simulink model "FlightSim"  (R2023a)
settings: Solver=VariableStepAuto  StartTime=0.0  StopTime=20  RelTol=1e-3
model workspace (MAT data in the model file):
  polyCoefficient = [1 1 1]
values from neighbouring files (symbols this model uses):
  airDensity = 1.2250    (= 1.225)    [FlightSimSetup.m]
  airKinematicViscosity = 1.48*10^-5    (= 1.48e-05)    [FlightSimSetup.m]
  AOA = deg2rad(0)    [FlightSimSetup.m]
  axialDistanceMACCGx = abs(finMACLeadingEdgeLocation) + finLeadingEdgeCGxlength    [finParameters.m]
  bodyDiameter = 2/100    (= 0.02)    [FlightSimSetup.m]
  bodyLength = 20/100    (= 0.2)    [FlightSimSetup.m]
  bodyLongitudinalInertia = bodyMass*( ((bodyDiameter/2).^2)/4 + ((bodyLength).^2)/12)    [InertiaParameters.m]
  bodyMass = 12/1000    (= 0.012)    [FlightSimSetup.m]
  bodyPlanformArea = bodyDiameter*bodyLength    (= 0.004)    [FlightSimSetup.m]
  bodyRoughness = 60*10^-6    (= 6e-05)    [FlightSimSetup.m]
  casingLongitudinalInertia = motorCasingMass/12*(3*(motorOuterRadius.^2+motorInnerRadius.^2)+motorLength.^2)    [InertiaParameters.m]
  cYaw = 0    [FlightSimSetup.m]
  dIdt = zeros(3)    [InertiaParameters.m]
  finArea = (finRootChord+finTailChord)*finSpan/2    [finParameters.m]
  finCantAngle = deg2rad(0)    [FlightSimSetup.m]
  finCentroidAxial = finSpan/3 * ( (finRootChord + 2*finTailChord)/(finRootChord + finTailChord) ) - backOffset    [finParameters.m]
  finCount = 4    [FlightSimSetup.m]
  finFrontSweepAngle = acos(dot(frontSweepAngleRefVector1, frontSweepAngleRefVector2) / (norm(frontSweepAngleRefVector1) * norm(frontSweepAngleRefVector2)))    [finParameters.m]
  finLeadingEdgeNoseConeDistance = bodyLength + noseConeHeight - finRootChord + backOffset    [finParameters.m]
  finLongitudinalInertia = finLongitudinalInertia * singleFinMass * finCount    [InertiaParameters.m]
  finMACLeadingEdgeLocation = abs( integral(finMACLeadingEdgeLocationFunction, 0, finSpan)/finArea )    [finParameters.m]
  finMACLength = integral(chordLengthFunction, 0, finSpan)/finArea    [finParameters.m]
  finMACNoseconeAxialDistance = finLeadingEdgeNoseConeDistance + finMACLeadingEdgeLocation+ 1/2*finMACLength    [finParameters.m]
  finMidChordSweepAngle = acos(dot(midChordAngleRefVector1, midChordAngleRefVector2) / (norm(midChordAngleRefVector1) * norm(midChordAngleRefVector2)))    [finParameters.m]
  finProfileType = 3    [FlightSimSetup.m]
  finRootChord = abs(P_frontRoot(2)-P_backRoot(2))    [finParameters.m]
  finSpan = abs(P_frontTrail(1)- P_frontRoot(1))    [finParameters.m]
  finTailChord = abs(P_frontTrail(2)-P_backTrail(2))    [finParameters.m]
  finThickness = 0.15/100    (= 0.0015)    [FlightSimSetup.m]
  finX_t = P_frontRoot(2) - P_frontTrail(2)    [finParameters.m]
  launchLugInnerDiameter = 0    [FlightSimSetup.m]
  launchLugLength = 0    [FlightSimSetup.m]
  launchLugOuterDiameter = 0    [FlightSimSetup.m]
  machNum = 0    [FlightSimSetup.m]
  motorCasingMass = motorInitialTotalMass - motorInitialPropMass    (= 0.071)    [FlightSimSetup.m]
  motorInitialPropMass = 83/1000    (= 0.083)    [FlightSimSetup.m]
  motorInnerRadius = motorOuterRadius - casingThickness    [InertiaParameters.m]
  motorLength = 156/1000    (= 0.156)    [FlightSimSetup.m]
  motorTotalImpulse = 129.9    [FlightSimSetup.m]
  noseConeHeight = 6/100    (= 0.06)    [FlightSimSetup.m]
  noseConeJoinAngle = pi/2 - atan(noseConeHeight/(bodyDiameter/2))    [FlightSimSetup.m]
  noseConeLongitudinalInertia = noseConeLongitudinalInertia*noseConeMass    [noseConeParameters.m]
  noseConeMass = 5/1000    (= 0.005)    [FlightSimSetup.m]
  noseConePlanformArea = (1/2)*bodyDiameter* noseConeHeight    [noseConeParameters.m]
  noseConeType = 8    [FlightSimSetup.m]
  noseConeVolume = (1/3)*noseConeHeight*(bodyDiameter/2)^2*pi    [noseConeParameters.m]
  noseConeWettedArea = pi*sqrt( (bodyDiameter/2)^2 + noseConeHeight^2)*bodyDiameter/2    [noseConeParameters.m]
  pitchCenterX = 0    [FlightSimSetup.m]
  pitchRate = 0    [FlightSimSetup.m]
  radialMACPosition = finMACSpanwisePosition + bodyDiameter/2    [finParameters.m]
  refArea = bodyDiameter^2*pi/4    (= 0.000314159265359)    [FlightSimSetup.m]
  rocketLength = (20+6)/100    (= 0.26)    [FlightSimSetup.m]
  rocketNonMotorMass = noseConeMass + bodyMass + totalFinMass    (= 0.021)    [FlightSimSetup.m]
  rocketRotationalInertia = bodyRotationalInertia + casingRotationalInertia + finRotationalInertia + noseConeLongitudinalInertia    [InertiaParameters.m]
  rocketVelocityMag = machNum*340.17    (= 0)    [FlightSimSetup.m]
  rollRate = 0    [FlightSimSetup.m]
  totalFinMass = singleFinMass * finCount    (= 0.004)    [FlightSimSetup.m]
  windAngle = 0    [FlightSimSetup.m]
  yawRate = 0    [FlightSimSetup.m]
libraries: shared6dof x1, aerolibanim x1, aerolibadyn x1, aerolibbdyn x1, sharedutil x1
(part 2 of 3)

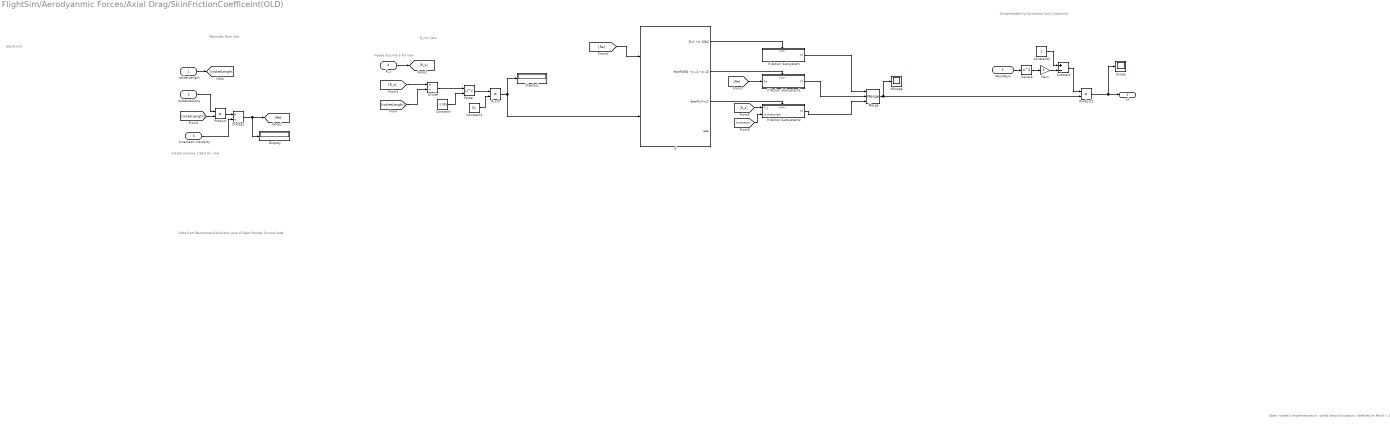
== system FlightSim/Aerodyanmic Forces/Axial Drag/SkinFrictionCoefficeint(OLD) ==
  note: Open rocket's implementation, works beyond subsonic (defined at Mach <.9)
  note: Code from BarrowmanCalculator.java of Open Rocket Source Code
  note: maybe assume 5 for now
  note: equations
  note: R_crit Calc
  note: Reynolds Num Calc
  note: maybe assume 1.5E-5 for now
  note: Compressability Correction (only Subsonic)
  rocketLength : Inport
  rocketVelocity : Inport  Port=2
  kinematic Viscosity : Inport  Port=3
  R_s : Inport  Port=4
  MachNum : Inport  Port=5
  Constant : Constant  Value=-1.039
  Constant1 : Constant  Value=51
  Constant2 : Constant
  Display : Display
  Display1 : Display
  Divide : Product  Inputs=*/
  Divide1 : Product  Inputs=*/
  Gain : Gain  Gain=.1
  If : If  NumInputs=2  IfExpression=u1 <= 10E4  ElseIfExpressions="10E4 <= u1 <= u2,  u1>u2"
  If Action Subsystem : Subsystem  TreatAsAtomicUnit=on
  If Action Subsystem1 : Subsystem  TreatAsAtomicUnit=on
  If Action Subsystem2 : Subsystem  TreatAsAtomicUnit=on
  Merge : Merge  Inputs=3
  Power : Math  Operator=pow
  Product : Product
  Product1 : Product
  R_crit : Product
  Scope : Scope
  Square : Math  Operator=square
  Subtract : Sum  Inputs=+-
  cfScope : Scope
  Cf : Outport
  wires:
  [R_s] -> Divide/1
  Divide/1 -> Power/1
  Constant/1 -> Power/2
  Power/1 -> R_crit/1
  Constant1/1 -> R_crit/2
  rocketLength/1 -> [rocketLength]
  R_crit/1 -> Display1/1, If/2
  [rocketLength] -> Divide/2
  [rocketLength] -> Product/2
  rocketVelocity/1 -> Product/1
  Product/1 -> Divide1/1
  kinematic Viscosity/1 -> Divide1/2
  Divide1/1 -> Display/1, [Re]
  R_s/1 -> [R_s]
  [Re] -> If/1
  If/1 -> If Action Subsystem/Action
  If/2 -> If Action Subsystem1/Action
  If Action Subsystem1/1(Cf) -> Merge/2
  If/3 -> If Action Subsystem2/Action
  If Action Subsystem2/1(Cf) -> Merge/3
  If Action Subsystem/1(Cf) -> Merge/1
  Merge/1 -> cfScope/1, Product1/2
  [Re] -> If Action Subsystem1/1(Re)
  [R_s] -> If Action Subsystem2/1(R_s)
  [rocketLength] -> If Action Subsystem2/2(rocketLength)
  MachNum/1 -> Square/1
  Square/1 -> Gain/1
  Gain/1 -> Subtract/2
  Constant2/1 -> Subtract/1
  Subtract/1 -> Product1/1
  Product1/1 -> Scope/1, Cf/1

== system FlightSim/Aerodyanmic Forces/Axial Drag/SkinFrictionCoefficient ==
  note: Open rocket's implementation, works beyond subsonic (defined at Mach <.9)
  note: Code from BarrowmanCalculator.java of Open Rocket Source Code
  note: equations
  note: Reynolds Num Calc
  note: maybe assume 1.5E-5 for now
  note: Compressability Correction (only Subsonic)
  note: Code from BarrowmanCalculator.java of Open Rocket Source Code, lines 601 to 632
  note: Roughness Comparison Body (Cf the same for Body, Fin, and nosecone)
  note: Code from BarrowmanCalculator.java of Open Rocket Source Code, lines 462-466
  rocketLength : Inport
  rocketVelocity : Inport  Port=2
  kinematic Viscosity : Inport  Port=3
  R_s : Inport  Port=4
  MachNum : Inport  Port=5
  Cf Value : Display
  Cf(R>R_Crit) : Product
  Constant : Constant  Value=1.48e-2
  Constant1 : Constant  Value=5.6
  Constant2 : Constant
  Constant3 : Constant
  Constant4 : Constant  Value=.2
  Constant5 : Constant  Value=0.032
  Display1 : Display
  Divide1 : Product  Inputs=*/
  Divide2 : Product  Inputs=*/
  Divide3 : Product  Inputs=*/
  Gain : Gain  Gain=.1
  Gain1 : Gain  Gain=1.5
  Log : Math  Operator=log
  Max : MinMax  Function=max  Inputs=2
  Power1 : Math  Operator=pow
  Product : Product
  Product1 : Product
  Product2 : Product
  Square : Math  Operator=square
  Square1 : Math  Operator=square
  Subtract : Sum  Inputs=+-
  Subtract1 : Sum  Inputs=+-
  Switch : Switch  Criteria=u2 > Threshold  Threshold=1e4  SaturateOnIntegerOverflow=off  InputSameDT=off
  reynolds Number : Display
  roughnessCorrection : Scope
  roughnessLimitedBody : Display
  Cf : Outport
  wires:
  rocketLength/1 -> [rocketLength]
  [rocketLength] -> Product/2
  rocketVelocity/1 -> Product/1
  Product/1 -> Divide1/1
  kinematic Viscosity/1 -> Divide1/2
  Divide1/1 -> Log/1, Switch/2, reynolds Number/1
  Switch/1 -> Product1/2
  MachNum/1 -> Square/1
  Square/1 -> Gain/1
  Gain/1 -> Subtract/2
  Constant2/1 -> Subtract/1
  Product1/1 -> Max/2, Cf Value/1
  Subtract1/1 -> Square1/1
  Constant1/1 -> Subtract1/2
  Square1/1 -> Divide2/2
  Log/1 -> Gain1/1
  Gain1/1 -> Subtract1/1
  Constant3/1 -> Divide2/1
  Constant/1 -> Switch/3
  Divide3/1 -> Power1/1
  Power1/1 -> Cf(R>R_Crit)/1
  Constant4/1 -> Power1/2
  Constant5/1 -> Cf(R>R_Crit)/2
  R_s/1 -> Divide3/1
  Cf(R>R_Crit)/1 -> Product2/1
  Subtract/1 -> Product1/1, roughnessCorrection/1, Product2/2
  Product2/1 -> Max/1, roughnessLimitedBody/1
  Max/1 -> Display1/1, Cf/1
  Divide2/1 -> Switch/1
  [rocketLength] -> Divide3/2

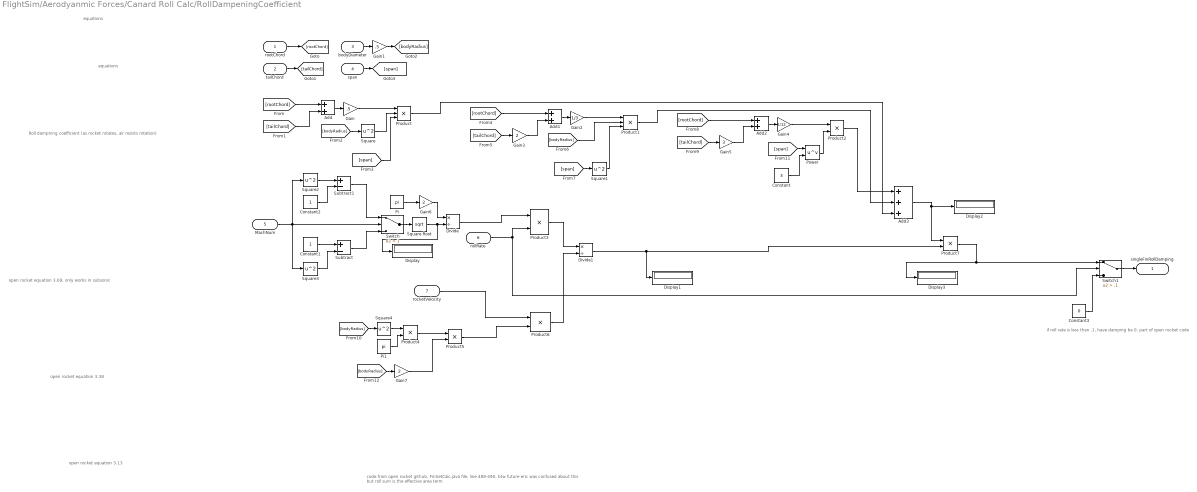
== system FlightSim/Aerodyanmic Forces/Canard Roll Calc/RollDampeningCoefficient ==
  note: if roll rate is less than .1, have damping be 0, part of open rocket code
  note: code from open rocket github, FinSetCalc.java file, line 488-490, btw future eric was confused about this but roll sum is the effective area term
  note: open rocket equation 3.38
  note: Roll dampning coefficient (as rocket rotates, air resists rotation)
  note: equations
  note: equations
  note: open rocket equation 3.13
  note: open rocket equation 3.69, only works in subsonic
  rootChord : Inport
  tailChord : Inport  Port=2
  bodyDiameter : Inport  Port=3
  span : Inport  Port=4
  MachNum : Inport  Port=5
  rollRate : Inport  Port=6
  rocketVelocity : Inport  Port=7
  Add : Sum
  Add1 : Sum
  Add2 : Sum
  Add3 : Sum  Inputs=+++
  Constant : Constant  Value=3
  Constant1 : Constant
  Constant2 : Constant
  Constant3 : Constant  Value=0
  Display{,1..3} (4 alike) : Display
  Divide : Product  Inputs=*/
  Divide1 : Product  Inputs=*/
  Gain : Gain  Gain=.5
  Gain1 : Gain  Gain=.5
  Gain2 : Gain  Gain=1/3
  Gain3 : Gain  Gain=2
  Gain4 : Gain  Gain=1/12
  Gain5 : Gain  Gain=3
  Gain6 : Gain  Gain=2
  Gain7 : Gain  Gain=2
  Pi : Constant  Value=pi  OutDataTypeStr=Inherit: Inherit via back propagation
  Pi1 : Constant  Value=pi  OutDataTypeStr=Inherit: Inherit via back propagation
  Power : Math  Operator=pow
  Product : Product  Inputs=3
  Product1 : Product  Inputs=3
  Product{2..7} (6 alike) : Product
  Square{,1..4} (5 alike) : Math  Operator=square
  Square Root : Sqrt
  Subtract : Sum  Inputs=+-
  Subtract1 : Sum  Inputs=+-
  Switch : Switch  Criteria=u2 > Threshold  Threshold=1  SaturateOnIntegerOverflow=off  InputSameDT=off
  Switch1 : Switch  Criteria=u2 > Threshold  Threshold=.1  SaturateOnIntegerOverflow=off  InputSameDT=off
  singleFinRollDamping : Outport
  wires:
  Add/1 -> Gain/1
  rootChord/1 -> [rootChord]
  tailChord/1 -> [tailChord]
  bodyDiameter/1 -> Gain1/1
  span/1 -> [span]
  [rootChord] -> Add/1
  [tailChord] -> Add/2
  Gain/1 -> Product/1
  Gain1/1 -> [bodyRadius]
  [bodyRadius] -> Square/1
  Square/1 -> Product/2
  [span] -> Product/3
  [tailChord] -> Gain3/1
  [rootChord] -> Add1/1
  Add1/1 -> Gain2/1
  Gain2/1 -> Product1/1
  Gain3/1 -> Add1/2
  [span] -> Square1/1
  Square1/1 -> Product1/3
  [bodyRadius] -> Product1/2
  [span] -> Power/1
  [tailChord] -> Gain5/1
  Gain5/1 -> Add2/2
  [rootChord] -> Add2/1
  Add2/1 -> Gain4/1
  Gain4/1 -> Product2/1
  Constant/1 -> Power/2
  Power/1 -> Product2/2
  Product2/1 -> Add3/1
  Product1/1 -> Add3/2
  Product/1 -> Add3/3
  Add3/1 -> Display2/1, Product7/1
  MachNum/1 -> Switch/2, Square3/1, Square2/1
  Constant1/1 -> Subtract/1
  Square3/1 -> Subtract/2
  Subtract/1 -> Switch/3
  Constant2/1 -> Subtract1/2
  Square2/1 -> Subtract1/1
  Subtract1/1 -> Switch/1
  rollRate/1 -> Product3/2, Switch1/2
  Pi/1 -> Gain6/1
  Gain6/1 -> Divide/1
  Divide/1 -> Product3/1
  [bodyRadius] -> Square4/1
  Square4/1 -> Product4/1
  Pi1/1 -> Product4/2
  Product4/1 -> Product5/1
  [bodyRadius] -> Gain7/1
  Gain7/1 -> Product5/2
  Product5/1 -> Product6/2
  rocketVelocity/1 -> Product6/1
  Product3/1 -> Divide1/1
  Product6/1 -> Divide1/2
  Divide1/1 -> Display1/1, Product7/2
  Product7/1 -> Switch1/1, Display3/1
  Switch/1 -> Square Root/1
  Square Root/1 -> Display/1, Divide/2
  Constant3/1 -> Switch1/3
  Switch1/1 -> singleFinRollDamping/1

== system FlightSim/Aerodyanmic Forces/Canard Roll Calc/RollForcingCoefficient ==
  note: radialMACSpan already has body radius considered
  note: equations
  bodyDiameter : Inport
  radialMACPosition : Inport  Port=2
  CNa : Inport  Port=3
  finCantAngle : Inport  Port=4
  finSpan : Inport  Port=5
  Add : Sum
  Add2 : Sum
  Add3 : Sum
  Constant5 : Constant
  Display{1..5} (5 alike) : Display
  Divide : Product  Inputs=*/
  Divide3 : Product  Inputs=*/  RndMeth=Zero  SaturateOnIntegerOverflow=on
  Gain : Gain  Gain=.5
  Product1 : Product
  Product2 : Product
  Product3 : Product
  singeFinRollForcing : Outport
  wires:
  radialMACPosition/1 -> Add/1, Display1/1
  bodyDiameter/1 -> Divide/2, Gain/1
  finCantAngle/1 -> Display3/1, Product2/2
  Divide/1 -> Display5/1, singeFinRollForcing/1
  Product2/1 -> Divide/1
  Product1/1 -> Product2/1
  Add/1 -> Product1/1
  CNa/1 -> Display2/1, Product3/1
  Divide3/1 -> Add3/2
  Gain/1 -> Add2/1, Divide3/1
  Constant5/1 -> Add3/1
  Add2/1 -> Divide3/2
  finSpan/1 -> Add2/2
  Product3/1 -> Product1/2
  Add3/1 -> Product3/2, Display4/1

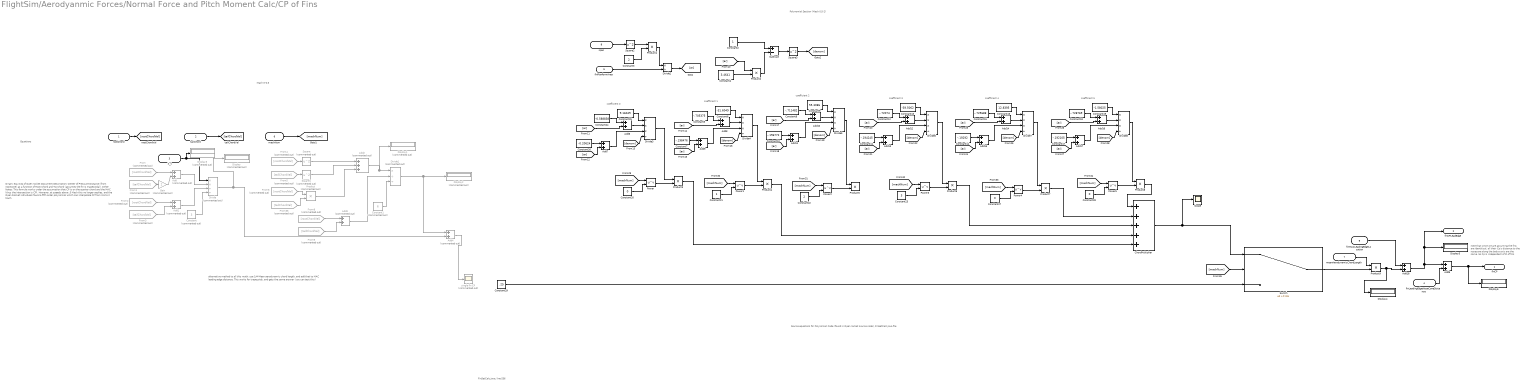
== system FlightSim/Aerodyanmic Forces/Normal Force and Pitch Moment Calc/CP of Fins ==
  note: FinSetCalc.java, line 536
  note: alternative method to all this math: use 1/4*Mean aerodynamic chord length, and add that to MAC leading edge distance. This works for trapezoids, and gets the same answer (you can test this)
  note: note that since we are assuming the fins are identitical, all their Cp's distance to the nosecone along the bodya axis are the same. So Cp is independent of # of fins
  note: Mach 0-0.5
  note: Source equations for Polynomail Code (found in Open rocket source code), FinSetCalc.java file
  note: Polynomial Section (Mach 0.5-2)
  note: coefficient 0
  note: Equations
  note: Origin: equ 3.34  of Open rocket Document
      |
      | Description: Center of Pressure Distance (from nosecone)
      | as a function of Root Chord and Tip Chord (assumes the fin is trapezoidal).
      |
      | Other Notes: This formula works under the assumption that CP is on the quarter chord
      | and the MAC (thus the intersection is CP). However, at speeds above .5 Mach this no longer applies, and the Open Rocket individuals found a fifth order polynomial which can interpolate CP from 0.5 to 2 Mach
  note: coefficient 1
  note: coefficient 2
  note: coefficient 3
  note: coefficient 4
  note: coefficient 5
  rootChord : Inport
  tailChord : Inport  Port=2
  X_t : Inport  Port=3
  finLeadingEdgeNoseConeDistance : Inport  Port=4
  finPlanformArea : Inport  Port=5
  machNum : Inport  Port=6
  meanAerodynamicChordLength : Inport  Port=7
  span : Inport  Port=8
  finMACLeadingEdgeLocation : Inport  Port=9
  Add{,1,3..4} (4 alike) : Sum  Commented=on
  Add{5..18} (14 alike) : Sum
  Add2 : Sum  Commented=on  Inputs=+++
  ChordMultiplier : Sum  Inputs=+++++
  Constant : Constant  Commented=on  Value=3
  Constant1 : Constant  Commented=on  Value=6
  Constant10 : Constant  Value=-.196772
  Constant11 : Constant  Value=-.194245
  Constant12 : Constant  Value=-.72074
  Constant13 : Constant  Value=-39.5062
  Constant14 : Constant  Value=-.19292
  Constant15 : Constant  Value=-.725688
  Constant16 : Constant  Value=12.8395
  Constant17 : Constant  Value=-.192105
  Constant18 : Constant  Value=-.728769
  Constant19 : Constant  Value=-1.58025
  Constant2 : Constant
  Constant20 : Constant  Value=0
  Constant21 : Constant
  Constant22 : Constant  Value=2
  Constant23 : Constant  Value=3
  Constant24 : Constant  Value=-0.588838
  Constant25 : Constant  Value=9.16049
  Constant26 : Constant  Value=-0.20624
  Constant27 : Constant  Value=0
  Constant28 : Constant  Value=0
  Constant29 : Constant  Value=.25
  Constant3 : Constant  Value=2
  Constant4 : Constant  Value=3.4641
  Constant5 : Constant  Value=-.705375
  Constant6 : Constant  Value=-31.6049
  Constant7 : Constant  Value=-.198476
  Constant8 : Constant  Value=-.711482
  Constant9 : Constant  Value=55.3086
  Display{,1..3} (4 alike) : Display  Commented=on
  Display4 : Display
  Display5 : Display
  Display6 : Display
  Divide : Product  Commented=on  Inputs=**//
  Divide1 : Product  Commented=on  Inputs=*//
  Divide2 : Product  Inputs=*/
  Divide{3..8} (6 alike) : Product  Inputs=***/
  From{,2,4,6,8} (5 alike) : From  Commented=on  GotoTag=rootChordVal
  From{1,3,5,9,36} (5 alike) : From  Commented=on  GotoTag=tailChordVal
  Gain : Gain  Commented=on  Gain=2
  Power{,1..5} (6 alike) : Math  Operator=pow
  Product : Product  Commented=on
  Product{1..9} (9 alike) : Product
  Scope : Scope
  Square : Math  Commented=on  Operator=square
  Square1 : Math  Commented=on  Operator=square
  Square2 : Math  Operator=square
  Square3 : Math  Operator=square
  Subtract : Sum  Inputs=+-
  Switch : Switch  Threshold=0.5  SaturateOnIntegerOverflow=off  InputSameDT=off
  single fin CP : Scope  Commented=on
  finCP : Outport
  finCPLeadEdge : Outport  Port=2
  wires:
  rootChord/1 -> [rootChordVal]
  tailChord/1 -> [tailChordVal]
  From/1 -> Add/1
  From1/1 -> Gain/1
  Gain/1 -> Add/2
  From2/1 -> Add1/1
  From3/1 -> Add1/2
  Add/1 -> Divide/2
  Add1/1 -> Divide/3
  Constant/1 -> Divide/4
  X_t/1 -> Display3/1, Divide/1
  From4/1 -> Square/1
  Square/1 -> Add2/1
  From5/1 -> Square1/1
  Square1/1 -> Add2/2
  From6/1 -> Product/1
  Product/1 -> Add2/3
  Add2/1 -> Display1/1, Divide1/1
  From9/1 -> Add3/2
  From8/1 -> Add3/1
  Add3/1 -> Divide1/2
  Constant1/1 -> Divide1/3
  Divide/1 -> Display/1, Add4/2
  Divide1/1 -> Add4/1, Display2/1
  Add4/1 -> single fin CP/1
  Constant3/1 -> Product1/2
  Square2/1 -> Product1/1
  Product1/1 -> Divide2/1
  finPlanformArea/1 -> Divide2/2
  span/1 -> Square2/1
  Divide2/1 -> [ar]
  machNum/1 -> [machNum]
  Constant4/1 -> Product2/2
  [ar] -> Product2/1
  Constant2/1 -> Subtract/1
  Product2/1 -> Subtract/2
  Subtract/1 -> Square3/1
  Square3/1 -> [denom]
  Constant25/1 -> Divide3/1
  Constant24/1 -> Add6/1
  [ar] -> Add6/2
  Constant26/1 -> Add7/1
  [ar] -> Add7/2
  Add7/1 -> Divide3/3
  Add6/1 -> Divide3/2
  [denom] -> Divide3/4
  [ar] -> Add9/2
  Constant7/1 -> Add9/1
  Add8/1 -> Divide4/2
  Constant6/1 -> Divide4/1
  Constant5/1 -> Add8/1
  [denom] -> Divide4/4
  Add9/1 -> Divide4/3
  [ar] -> Add8/2
  Add11/1 -> Divide5/3
  Constant10/1 -> Add11/1
  [denom] -> Divide5/4
  Add10/1 -> Divide5/2
  [ar] -> Add10/2
  [ar] -> Add11/2
  Constant8/1 -> Add10/1
  Constant9/1 -> Divide5/1
  [ar] -> Add12/2
  [denom] -> Divide6/4
  Constant11/1 -> Add13/1
  [ar] -> Add13/2
  Constant12/1 -> Add12/1
  Constant13/1 -> Divide6/1
  Add13/1 -> Divide6/3
  Add12/1 -> Divide6/2
  [ar] -> Add14/2
  Constant14/1 -> Add15/1
  Constant15/1 -> Add14/1
  [denom] -> Divide7/4
  Add14/1 -> Divide7/2
  Add15/1 -> Divide7/3
  [ar] -> Add15/2
  Constant16/1 -> Divide7/1
  Constant19/1 -> Divide8/1
  [ar] -> Add16/2
  Add17/1 -> Divide8/3
  [ar] -> Add17/2
  [denom] -> Divide8/4
  Add16/1 -> Divide8/2
  Constant17/1 -> Add17/1
  Constant18/1 -> Add16/1
  Divide3/1 -> Product3/1
  [machNum] -> Power/1
  Constant20/1 -> Power/2
  Power/1 -> Product3/2
  [machNum] -> Power1/1
  Constant21/1 -> Power1/2
  Power1/1 -> Product4/2
  Divide4/1 -> Product4/1
  [machNum] -> Power2/1
  Constant22/1 -> Power2/2
  Power2/1 -> Product5/2
  Divide5/1 -> Product5/1
  [machNum] -> Power3/1
  Constant23/1 -> Power3/2
  Power3/1 -> Product6/2
  Divide6/1 -> Product6/1
  [machNum] -> Power4/1
  Constant27/1 -> Power4/2
  Power4/1 -> Product7/2
  Divide7/1 -> Product7/1
  [machNum] -> Power5/1
  Constant28/1 -> Power5/2
  Power5/1 -> Product8/2
  Divide8/1 -> Product8/1
  Product8/1 -> ChordMultiplier/1
  Product7/1 -> ChordMultiplier/2
  Product3/1 -> ChordMultiplier/5
  Product4/1 -> ChordMultiplier/4
  Product6/1 -> ChordMultiplier/3
  [machNum] -> Switch/2
  ChordMultiplier/1 -> Switch/1, Scope/1
  meanAerodynamicChordLength/1 -> Product9/1
  Switch/1 -> Product9/2
  Add18/1 -> finCPLeadEdge/1, Display5/1, Add5/1
  finLeadingEdgeNoseConeDistance/1 -> Add5/2
  Add5/1 -> Display6/1, finCP/1
  From36/1 -> Product/2
  finMACLeadingEdgeLocation/1 -> Add18/1
  Constant29/1 -> Switch/3
  Product9/1 -> Add18/2, Display4/1

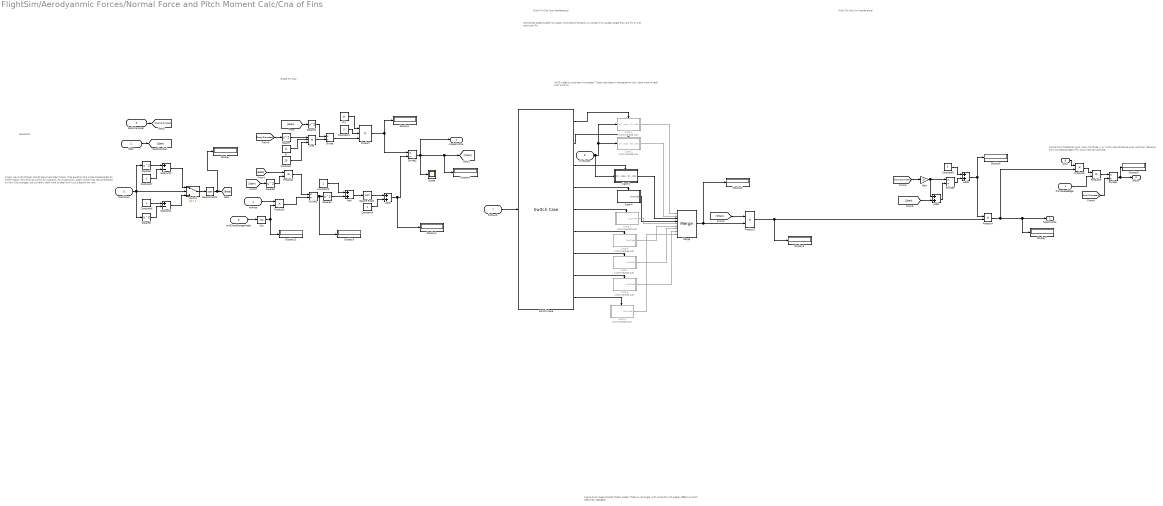
== system FlightSim/Aerodyanmic Forces/Normal Force and Pitch Moment Calc/Cna of Fins ==
  note: comes from finSetCalc.java, lines 179 (finds x, or in this case distance axial (vertical) distance from Cp leading edge of fin. Also lines 207 and 209
  note: NOTE: Need to consider wind angle?? thats the theta in the equation, but i dont want to deal with wind so...
  note: Origin: equ 3.40  of Open rocket Document
      |
      | Description: CNa equation of a single trapezopidal fin
      |
      | Other Notes: This formual works for subsonic. For supersonic, Open rockert has documentation on how CNa changes, but currently dont want to deal with it so subsonic for now
  note: Equations
  note: Single Fin CNa
  note: Multi Fin CNa (w/ interference)
  note: Somewhat questionable roll angle, since documentation is unclear if its largest angle from any fin or one particular fin
  note: FIgure From Open Rocket Thesis
      |
      | Notes: Theta is roll angle, with more fins roll angle's effect on pitch becomes negligble
  note: Multi Fin CNa (pre interference)
  finCnaLeadEdge : Inport
  Span : Inport  Port=2
  bodyDiameter : Inport  Port=3
  finArea : Inport  Port=4
  machNum : Inport  Port=5
  midChordSweepAngle : Inport  Port=6
  finCount : Inport  Port=7
  windAngle : Inport  Port=8
  AOA : Inport  Port=9
  Add{,1..3} (4 alike) : Sum
  Aref : Product  Inputs=3
  Case 1 : Subsystem  Commented=on  TreatAsAtomicUnit=on
  Case 2 : Subsystem  Commented=on  TreatAsAtomicUnit=on
  Case 3 : Subsystem  TreatAsAtomicUnit=on
  Case 4 : Subsystem  TreatAsAtomicUnit=on
  Case 5 : Subsystem  Commented=on  TreatAsAtomicUnit=on
  Case 6 : Subsystem  Commented=on  TreatAsAtomicUnit=on
  Case 7 : Subsystem  Commented=on  TreatAsAtomicUnit=on
  Case 8 : Subsystem  Commented=on  TreatAsAtomicUnit=on
  Case 9 : Subsystem  Commented=on  TreatAsAtomicUnit=on
  Constant : Constant  Value=.25
  Constant1 : Constant  Value=2
  Constant{3..7} (5 alike) : Constant
  Cos : Trigonometry  Operator=cos
  Display{,1..9,11} (11 alike) : Display
  Divide{,1..2,4} (4 alike) : Product  Inputs=*/
  Divide3 : Product  Inputs=*/  RndMeth=Zero  SaturateOnIntegerOverflow=on
  Gain : Gain  Gain=.5
  Merge : Merge  Inputs=9
  Pi : Constant  Value=pi  OutDataTypeStr=Inherit: Inherit via back propagation
  Pi1 : Constant  Value=pi  OutDataTypeStr=Inherit: Inherit via back propagation
  Product : Product  Inputs=3
  Product{1..6} (6 alike) : Product
  Scope : Scope
  Square{,1,3..6} (6 alike) : Math  Operator=square
  Square Root1 : Sqrt
  Square Root2 : Sqrt
  Subtract1 : Sum  Inputs=+-
  Subtract2 : Sum  Inputs=+-
  Switch : Switch  Criteria=u2 > Threshold  Threshold=1  SaturateOnIntegerOverflow=off  InputSameDT=off
  Switch Case : SwitchCase  CaseConditions={1,2,3,4,5,6,7,8}
  singleFinCNa : Outport
  totalFinCNa : Outport  Port=2
  finCm : Outport  Port=3
  wires:
  Span/1 -> [Span]
  Constant/1 -> Aref/3
  bodyDiameter/1 -> [bodyDiameter]
  Square/1 -> Aref/1
  Pi/1 -> Aref/2
  [Span] -> Square1/1
  Square1/1 -> Divide/1
  Aref/1 -> Divide/2
  Pi1/1 -> Product/1
  Constant1/1 -> Product/2
  Divide/1 -> Product/3
  [Span] -> Square3/1
  [Beta] -> Product1/1
  Square3/1 -> Product1/2
  Product1/1 -> Divide1/1
  finArea/1 -> Product2/1
  midChordSweepAngle/1 -> Cos/1
  Cos/1 -> Product2/2, Display11/1
  Product2/1 -> Divide1/2
  Divide1/1 -> Display3/1, Square4/1
  Constant3/1 -> Add/1
  Square4/1 -> Add/2
  Add/1 -> Square Root1/1
  Constant4/1 -> Add1/2
  Square Root1/1 -> Add1/1
  Product/1 -> Divide2/1, Display1/1
  Add1/1 -> Display2/1, Divide2/2
  Divide2/1 -> Display4/1, [CNa1], Scope/1, singleFinCNa/1
  finCount/1 -> Switch Case/1
  Switch Case/1 -> Case 1/Action
  windAngle/1 -> Case 3/1(rollAngle), Case 1/1(rollAngle), Case 2/1(rollAngle)
  Switch Case/2 -> Case 2/Action
  Switch Case/3 -> Case 3/Action
  Switch Case/4 -> Case 4/Action
  Switch Case/5 -> Case 5/Action
  Switch Case/9 -> Case 9/Action
  Switch Case/8 -> Case 8/Action
  Switch Case/7 -> Case 7/Action
  Switch Case/6 -> Case 6/Action
  Case 1/1(CNaTotal) -> Merge/1
  Case 2/1(CNaTotal) -> Merge/2
  Case 3/1(CnaTotal) -> Merge/3
  Case 4/1(CnaTotal) -> Merge/4
  Case 5/1(CnaTotal) -> Merge/5
  Case 6/1(CnaTotal) -> Merge/6
  Case 7/1(CnaTotal) -> Merge/7
  Case 8/1(CnaTotal) -> Merge/8
  Case 9/1(CnaTotal) -> Merge/9
  [CNa1] -> Product3/1
  Merge/1 -> Display5/1, Product3/2
  [bodyDiameter] -> Square/1
  [bodyDiameter] -> Gain/1
  Gain/1 -> Add2/1, Divide3/1
  [Span] -> Add2/2
  Divide3/1 -> Add3/2
  Add2/1 -> Divide3/2
  Constant5/1 -> Add3/1
  Product3/1 -> Display8/1, Product4/2
  Subtract2/1 -> Switch/1
  Square6/1 -> Subtract1/2
  Square5/1 -> Subtract2/1
  Constant7/1 -> Subtract2/2
  machNum/1 -> Square5/1, Switch/2, Square6/1
  Subtract1/1 -> Switch/3
  Constant6/1 -> Subtract1/1
  Switch/1 -> Square Root2/1
  Square Root2/1 -> Display/1, [Beta]
  Product4/1 -> Display7/1, totalFinCNa/1, Product6/2
  finCnaLeadEdge/1 -> Product5/2
  Product5/1 -> Divide4/1
  [bodyDiameter] -> Divide4/2
  Divide4/1 -> Display9/1, finCm/1
  AOA/1 -> Product6/1
  Product6/1 -> Product5/1
  Add3/1 -> Product4/1, Display6/1

== system FlightSim/Aerodyanmic Forces/Normal Force and Pitch Moment Calc/DampingCalculations ==
  velocity : Inport
  FinDampingMultiplier : Inport  Port=2
  bodyDampingMultiplier : Inport  Port=3
  pitchRate : Inport  Port=4
  yawRate : Inport  Port=5
  Add : Sum
  Display : Display
  Display1 : Display
  Divide : Product  Inputs=*/
  Divide1 : Product  Inputs=*/
  Gain2 : Gain  Gain=3
  Product : Product
  Product1 : Product
  Square : Math  Operator=square
  Square1 : Math  Operator=square
  pitchDampingMoment : Outport
  yawDampingMoment : Outport  Port=2
  wires:
  bodyDampingMultiplier/1 -> Add/1
  FinDampingMultiplier/1 -> Add/2
  Add/1 -> Display1/1, Gain2/1
  pitchRate/1 -> Display/1, Divide/1
  velocity/1 -> Divide/2, Divide1/2
  yawRate/1 -> Divide1/1
  Divide/1 -> Square/1
  Divide1/1 -> Square1/1
  Gain2/1 -> Product1/2, Product/1
  Product/1 -> yawDampingMoment/1
  Square1/1 -> Product/2
  Square/1 -> Product1/1
  Product1/1 -> pitchDampingMoment/1

== system FlightSim/Aerodyanmic Forces/Normal Force and Pitch Moment Calc/FinPitchDampingMoment ==
  note: Open rocket BarrowmanCalculator.Java file, lines 973-985
  note: Origin: equation 3.6 Open Rocket
      |
      | Description: dampening moment of Fins
  note: Equations
  note: Open Rocket finSetCalc.Java file, lines 244-246
  finPlanformArea : Inport
  finNum : Inport  Port=2
  bodyDiameter : Inport  Port=3
  Constant : Constant  Value=4
  Constant1 : Constant  Value=3
  Constant2 : Constant  Value=refArea
  Constant3 : Constant  Value=0.6
  Constant5 : Constant  Value=finMACNoseconeAxialDistance
  Display : Display
  Display1 : Display
  Power : Math  Operator=pow
  Product{,1..3} (4 alike) : Product
  Switch : Switch  Threshold=4  SaturateOnIntegerOverflow=off  InputSameDT=off
  multiplier : Product  Inputs=*/
  finDampingMultiplier : Outport
  wires:
  finNum/1 -> Switch/3, Switch/2
  Constant/1 -> Switch/1
  Switch/1 -> Product/2
  finPlanformArea/1 -> Product/1
  Constant1/1 -> Power/2
  Power/1 -> Product1/2
  Product/1 -> Product1/1
  bodyDiameter/1 -> Product2/1
  Product1/1 -> Product3/1
  Constant3/1 -> Product3/2
  Product2/1 -> multiplier/2
  Product3/1 -> multiplier/1
  multiplier/1 -> finDampingMultiplier/1, Display/1
  Constant5/1 -> Display1/1, Power/1
  Constant2/1 -> Product2/2

== system FlightSim/Aerodyanmic Forces/Normal Force and Pitch Moment Calc/Normal, Cp,Pitch of Nosecone ==
  note: this implementation for CNa of nosecone is noticably different than equation 3.19, this is becuase this implementatio also involves calculating the lift of the nosecone. First branch implenets equation 3.19, while the bottom branch with the sublock calculates lift and then the CNa contributions of both are added together. one thing to note is this assumes the Cp of lift = Cp in 3.28.
  note: simplified version of eq 3.25 with A(l) = Aref, and l is height of nosecone
  note: assumes nosecone base diameter is rocket body diameter (since no shoulder). Also, A(0) is just the tip of nosecone, so 0. Therefore, Aref = A(l)
  note: Equations
  note: Origin: equ 3.19  of Open rocket Document
      |
      | Description: CNa Calculation for Axially Symmetric Bodies
  note: Origin: equ 3.25  of Open rocket Document
      |
      | Description: CMa Calculation for Axially Symmetric Bodies
  note: Origin: equ 3.28  of Open rocket Document
      |
      | Description: CP calculations, based on the 2 above equations
  note: makes sure that there is no divide by 0 error when AOA = 0,
      | and using small angle approximation when AOA = 0, sin(AOA)/AOA = 1
  note: simplified version of eq 3.28 with A(l) = Aref, and l is height of nosecone
  note: Text from Centuri Engineering Company Technical report: https://www.spacemodeling.org/jimz/manuals/tir-33.pdf
      | Validates that nosecone CNa is almost always 2, which the following eequation would get
  note: Figure from Centuri Engineering Company Technical report: https://www.spacemodeling.org/jimz/manuals/tir-33.pdf
      | Check if equations get values similar to this
  note: in this line of code from SymmetricComponentCalc.java (lines 137-40), we see them calculate CNa using equation 3.19, and then averaging it with the lift of the part. (in this context, average adds the CNa's together), seen in line 290 of Coordinate.java
  bodyDiameter : Inport
  noseconeVolume : Inport  Port=2
  noseconeHeight : Inport  Port=3
  AOA : Inport  Port=4
  noseConePlanformArea : Inport  Port=5
  Add : Sum
  Aref And A(l) : Product
  Constant3 : Constant
  Display{,1..4} (5 alike) : Display
  Divide : Product  Inputs=*/
  Divide1 : Product  Inputs=*/
  Divide3 : Product  Inputs=*/
  Gain : Gain  Gain=2
  Gain1 : Gain  Gain=.5
  Pi : Constant  Value=pi  OutDataTypeStr=Inherit: Inherit via back propagation
  Product : Product
  Scope1 : Scope
  Sin1 : Trigonometry
  Square : Math  Operator=square
  Subtract : Sum  Inputs=+-
  Switch1 : Switch  Criteria=u2 > Threshold  Threshold=0.001  SaturateOnIntegerOverflow=off  InputSameDT=off
  noseConeLiftFactor : Subsystem
  noseconeCNa : Outport
  noseconeCMa : Outport  Port=2
  noseconeCp : Outport  Port=3
  wires:
  Square/1 -> Aref And A(l)/1
  Pi/1 -> Aref And A(l)/2
  Sin1/1 -> Divide3/1
  AOA/1 -> noseConeLiftFactor/1(AOA), Sin1/1, Divide3/2, Switch1/2
  Divide3/1 -> Switch1/1
  Constant3/1 -> Switch1/3
  Gain/1 -> Add/1, Product/2
  noseconeVolume/1 -> Display1/1, Divide1/1
  noseconeHeight/1 -> Subtract/1, Display2/1
  Divide1/1 -> Subtract/2
  Divide/1 -> Product/1
  Switch1/1 -> Gain/1
  Product/1 -> Scope1/1, noseconeCMa/1
  Aref And A(l)/1 -> Divide1/2
  [bodyDiameter] -> Gain1/1
  Gain1/1 -> Square/1
  Subtract/1 -> noseconeCp/1, Display3/1, Divide/1
  bodyDiameter/1 -> [bodyDiameter]
  [bodyDiameter] -> Divide/2
  [bodyDiameter] -> noseConeLiftFactor/3(bodyDiameter)
  noseConePlanformArea/1 -> noseConeLiftFactor/2(noseConePlanformArea)
  noseConeLiftFactor/1(noseConeLift) -> Add/2, Display4/1
  Add/1 -> Display/1, noseconeCNa/1

== system FlightSim/Aerodyanmic Forces/Normal Force and Pitch Moment Calc/Normal, Cp,Pitch of Rocket Body ==
  note: note: this is slightly different than the equation (notably the sin%2 part). That is because implementation in OPen rocket is different, as shown below from SymmetricComponentCalc.java line 180
  note: Equations
  note: Origin: equ 3.19  of Open rocket Document
      |
      | Description: CNa Calculation for Axially Symmetric Bodies
      |
      | Other notes: we don't use this equation for rocket body since A(0) = A(L) assuming
      | its cylindrial
  note: Origin: equ 3.26  of Open rocket Document
      |
      | Description: CNa due to Body Lift, K is a constant roughly
      | equal to 1.1
  note: Origin: equ 3.27 of Open rocket Document
      |
      | Description: Center of Pressure Location (relative to nose cone) from lift, f
      | for Cylinder it is just located at the center of the body
  note: makes sure that there is no divide by 0 error when AOA = 0,
      | and using small angle approximation when AOA = 0, sin(AOA)/AOA = 1
  bodyLength : Inport
  bodyDiameter : Inport  Port=2
  NoseconeHeight : Inport  Port=3
  AOA : Inport  Port=4
  APlan : Product
  Aref : Product  Inputs=3
  CNa : Product  Inputs=3
  Constant : Constant  Value=.25
  Constant3 : Constant  Value=0
  Cp : Sum
  Display{,1..3} (4 alike) : Display
  Divide : Product  Inputs=*/
  Divide3 : Product  Inputs=*/
  Gain : Gain  Gain=0.5
  K : Constant  Value=1.1
  Pi : Constant  Value=pi  OutDataTypeStr=Inherit: Inherit via back propagation
  Scope1 : Scope
  Sin1 : Trigonometry
  Square : Math  Operator=square
  Square1 : Math  Operator=square
  Switch1 : Switch  Criteria=u2 > Threshold  Threshold=0.001  SaturateOnIntegerOverflow=off  InputSameDT=off
  BodyCNa : Outport
  BodyCp : Outport  Port=2
  wires:
  bodyLength/1 -> Gain/1, APlan/1
  bodyDiameter/1 -> APlan/2, Square/1
  Constant/1 -> Aref/3
  Pi/1 -> Aref/2
  Square/1 -> Aref/1
  APlan/1 -> Display1/1, Divide/1
  Aref/1 -> Display2/1, Divide/2
  Divide/1 -> Display/1, CNa/1
  K/1 -> CNa/2
  Gain/1 -> Cp/1
  NoseconeHeight/1 -> Cp/2
  CNa/1 -> Display3/1, BodyCNa/1
  Cp/1 -> Scope1/1, BodyCp/1
  Switch1/1 -> CNa/3
  Divide3/1 -> Switch1/1
  Constant3/1 -> Switch1/3
  Sin1/1 -> Square1/1
  AOA/1 -> Sin1/1, Switch1/2, Divide3/2
  Square1/1 -> Divide3/1

== system FlightSim/Aerodyanmic Forces/Normal Force and Pitch Moment Calc/finalRocketCmCyawCalc ==
  note: combination of above lines, from BarrowmanCalculator.java, lines 206-207 and lines 944-945
  RocketCmNonDamping : Inport
  PitchDampingMoment : Inport  Port=2
  cYaw : Inport  Port=3
  PitchcYaw : Inport  Port=4
  Constant : Constant  Value=0
  Constant1 : Constant  Value=0
  Max : MinMax  Function=max  Inputs=2
  Max1 : MinMax  Function=max  Inputs=2
  Subtract : Sum  Inputs=+-
  Subtract1 : Sum  Inputs=+-
  rocketCm : Outport
  rocketcYaw : Outport  Port=2
  wires:
  PitchDampingMoment/1 -> Subtract/2
  RocketCmNonDamping/1 -> Subtract/1
  Max/1 -> rocketCm/1
  Constant/1 -> Max/2
  Subtract/1 -> Max/1
  Max1/1 -> rocketcYaw/1
  Constant1/1 -> Max1/2
  Subtract1/1 -> Max1/1
  cYaw/1 -> Subtract1/1
  PitchcYaw/1 -> Subtract1/2

== system FlightSim/Aerodyanmic Forces/Normal Force and Pitch Moment Calc/rocketBodyPitchDamping ==
  note: Note, cgx seems like 0? not sure why it is but okay
  note: relative to the base of the rocket
  note: note: r_t is the average radius of the body. Since we are just assuming a cylinderical body (no shoulder and botail), R_t = 1/2*d, which therefore simplifies the equation
  note: Equations
  note: Code from Open Rocket github, BarrowmanCalculator.Java, lines 954-974
  bodyLength : Inport
  CGAxialPosition : Inport  Port=2
  Add : Sum
  CacheLength : Sum
  Constant : Constant  Value=noseConeHeight
  Constant1 : Constant  Value=bodyDiameter  VectorParams1D=off
  Constant2 : Constant  Value=bodyLength
  Constant3 : Constant  Value=noseConePlanformArea
  Constant4 : Constant  Value=bodyPlanformArea
  Constant5 : Constant  Value=refArea
  Constant6 : Constant  Value=4
  Constant7 : Constant  Value=4
  Constant8 : Constant  Value=pitchCenterX
  Display{1..6} (6 alike) : Display
  Divide2 : Product  Inputs=*/
  Gain1 : Gain  Gain=.275
  Mul : Product
  Power : Math  Operator=pow
  Power1 : Math  Operator=pow
  Product1 : Product
  Subtract : Sum  Inputs=+-
  cacheArea : Sum
  cacheDiameter : Product  Inputs=*/
  bodyDampingMultiplier : Outport
  wires:
  bodyLength/1 -> ?
  Gain1/1 -> Divide2/1
  Constant1/1 -> Product1/2
  Product1/1 -> Divide2/2
  Divide2/1 -> Mul/1, Display1/1
  Constant2/1 -> CacheLength/1
  Constant/1 -> CacheLength/2
  Constant3/1 -> Display5/1, cacheArea/1
  Constant4/1 -> Display6/1, cacheArea/2
  cacheArea/1 -> Display3/1, cacheDiameter/1
  CacheLength/1 -> Subtract/1, cacheDiameter/2
  cacheDiameter/1 -> Display2/1, Gain1/1
  Constant5/1 -> Product1/1
  Constant6/1 -> Power/2
  Constant8/1 -> Subtract/2, Power1/1
  Constant7/1 -> Power1/2
  Power/1 -> Add/2
  Subtract/1 -> Power/1
  Power1/1 -> Add/1
  Add/1 -> Mul/2
  Mul/1 -> bodyDampingMultiplier/1, Display4/1

== system FlightSim/Aerodyanmic Forces/Normal Force and Pitch Moment Calc/rocketCMClalculation ==
  note: lines 144 and 145 in symmetricComponentCalc.java
  note: rearranges this equation to calculate Cm
  note: lines 234 in symmetricComponentCalc.java
  rocketCp : Inport
  rocketCn : Inport  Port=2
  rocketDiameter : Inport  Port=3
  Divide : Product  Inputs=*/
  Product : Product
  rocketCmNonDamping : Outport
  wires:
  rocketCn/1 -> Product/2
  rocketCp/1 -> Product/1
  Divide/1 -> rocketCmNonDamping/1
  Product/1 -> Divide/1
  rocketDiameter/1 -> Divide/2

== system FlightSim/Aerodyanmic Forces/Normal Force and Pitch Moment Calc/totalCP,CNa, Cn,Calculation ==
  note: note: body CP prob wrong, but body Cna is 0 so doesn't matter
  note: from openrocket document
  note: equations
  note: from https://www.spacemodeling.org/jimz/manuals/tir-33.pdf.
      |
      | Other Notes: There is a discrepency in notation between this document and Open Rocket. In Open Rocket, CNa is the derivative of normal force coefficient (which needs to be multiplied by AOA to get normal force), while in this document CNa is just the normal force coefficient
  bodyCNa : Inport
  bodyCP : Inport  Port=2
  noseconeCna : Inport  Port=3
  noseconeCp : Inport  Port=4
  finCP : Inport  Port=5
  totalFinCNa : Inport  Port=6
  nonCanardAOA : Inport  Port=7
  Add : Sum  Inputs=+++
  Add1 : Sum  Inputs=+++
  Display{,1..5} (6 alike) : Display
  Divide : Product  Inputs=*/
  Product{,1..3} (4 alike) : Product
  rocketCp : Outport
  nonCanardCN : Outport  Port=2
  totalCNa : Outport  Port=3
  wires:
  totalFinCNa/1 -> Display2/1, Product2/1, Add/3
  noseconeCna/1 -> Product1/2, Add/2
  bodyCNa/1 -> Display3/1, Product/2, Add/1
  Add/1 -> Product3/1, Display1/1, totalCNa/1, Divide/2
  bodyCP/1 -> Product/1, Display4/1
  noseconeCp/1 -> Product1/1
  finCP/1 -> Product2/2
  Product/1 -> Add1/1
  Product1/1 -> Add1/2
  Product2/1 -> Add1/3
  Add1/1 -> Divide/1
  Divide/1 -> Display5/1, rocketCp/1
  nonCanardAOA/1 -> Product3/2
  Product3/1 -> Display/1, nonCanardCN/1

== system FlightSim/Forces and Rotations/CG Calculations/Body Frame CG ==
  note: To find mass of rocket, take rocket non motor mass + motor shell mass + motor propellant mass at that instance
  rocketNonMotorMass : Inport
  motorCasingMass : Inport  Port=2
  motorPropMass : Inport  Port=3
  m_dot : Inport  Port=4
  Add : Sum  Inputs=+++
  Cg Body Axis : Scope
  Estimate Center of Gravity : aerolibbdyn/Estimate Center of Gravity  fmass=0.175  emass=0.092  fcg=[0.08148;0;0]  ecg=[0.0846195652173913;0;0]
  Scope1 : Scope
  Scope2 : Scope
  bodyFrameCG : Outport
  bodyFrameCG_dot : Outport  Port=2
  wires:
  rocketNonMotorMass/1 -> Add/1
  motorCasingMass/1 -> Add/2
  motorPropMass/1 -> Add/3
  m_dot/1 -> Estimate Center of Gravity/2, Scope1/1
  Estimate Center of Gravity/1 -> Cg Body Axis/1, bodyFrameCG/1
  Add/1 -> Scope2/1, Estimate Center of Gravity/1
  Estimate Center of Gravity/2 -> bodyFrameCG_dot/1

== system FlightSim/Aerodyanmic Forces/Axial Drag/Body Pressure Drag/BaseDrag ==
  note: Equations
  MachNum : Inport
  Add : Sum
  Constant : Constant  Value=.12
  Constant1 : Constant  Value=.25
  Divide : Product  Inputs=*/
  Gain : Gain  Gain=.13
  Square : Math  Operator=square
  Switch : Switch  Criteria=u2 > Threshold  Threshold=1  SaturateOnIntegerOverflow=off  InputSameDT=off
  baseDragCD : Outport
  wires:
  MachNum/1 -> Square/1, Divide/2, Switch/2
  Square/1 -> Gain/1
  Gain/1 -> Add/2
  Constant/1 -> Add/1
  Add/1 -> Switch/3
  Constant1/1 -> Divide/1
  Divide/1 -> Switch/1
  Switch/1 -> baseDragCD/1

== system FlightSim/Aerodyanmic Forces/Axial Drag/Body Pressure Drag/FinCD ==
  note: Equations
  note: finProfileType is a string with 1 of 3 values. 1 for "Round", 2 for "Airfoil", or 3 for "Square".
  finProfileType : Inport
  finFrontSweepAngle : Inport  Port=2
  MachNum : Inport  Port=3
  stagnationPressure : Inport  Port=4
  "Base Drag " : Inport  Port=5
  AirfoilShape : Subsystem  TreatAsAtomicUnit=on
  Display1 : Display
  Merge : Merge  Inputs=3
  RoundShape : Subsystem  TreatAsAtomicUnit=on
  SquareShape : Subsystem  TreatAsAtomicUnit=on
  Switch Case : SwitchCase  CaseConditions={1,2,3}
  singleFinPressureCD : Outport
  wires:
  finProfileType/1 -> Switch Case/1
  Switch Case/1 -> RoundShape/Action
  Switch Case/2 -> AirfoilShape/Action
  Switch Case/3 -> SquareShape/Action
  MachNum/1 -> [machNum]
  finFrontSweepAngle/1 -> [finFrontSweepAngle]
  "Base Drag "/1 -> [baseDrag]
  [finFrontSweepAngle] -> AirfoilShape/1(frontSweepAngle)
  [finFrontSweepAngle] -> SquareShape/1(frontSweepAngle)
  [baseDrag] -> RoundShape/1(baseDrag)
  [machNum] -> AirfoilShape/2(machNum)
  [baseDrag] -> SquareShape/3(baseDrag)
  stagnationPressure/1 -> SquareShape/2(stagnationPressure)
  RoundShape/1(roundFinPressureCD) -> Merge/1
  AirfoilShape/1(airfoilFinPressureCD) -> Merge/2
  SquareShape/1(squareFinPressureCD) -> Merge/3
  Merge/1 -> Display1/1, singleFinPressureCD/1
  [machNum] -> RoundShape/3(machNum)
  [finFrontSweepAngle] -> RoundShape/2(frontSweepAngle)

== system FlightSim/Aerodyanmic Forces/Axial Drag/Body Pressure Drag/Parasitic Drag ==
  note: Equations
  CdStag : Inport
  launchLugLength : Inport  Port=2
  launchLugOuterDiameter : Inport  Port=3
  CdParasidic : Scope
  Constant : Constant  Value=1.3
  Constant1 : Constant
  Display : Display
  Divide : Product  Inputs=*/
  Gain : Gain  Gain=.3
  Max : MinMax  Function=max  Inputs=2
  Product : Product
  Subtract : Sum  Inputs=+-
  cdParasidic : Outport
  wires:
  launchLugLength/1 -> Divide/1
  launchLugOuterDiameter/1 -> Divide/2
  Constant/1 -> Subtract/1
  Divide/1 -> Gain/1
  Gain/1 -> Subtract/2
  Subtract/1 -> Max/1
  Constant1/1 -> Max/2
  CdStag/1 -> Display/1, Product/1
  Max/1 -> Product/2
  Product/1 -> cdParasidic/1, CdParasidic/1

== system FlightSim/Aerodyanmic Forces/Axial Drag/Body Pressure Drag/noseConeCD ==
  note: Text From open Rocket Document. This only really works at subsonic Speeds
  note: defined a variable with numeric inputs. 1 -7 correspond with the shapes on the graph. 8 means there is a join angle, which is provided separately (assumed 0 if no joint angle).
  noseConeType : Inport
  joinAngle : Inport  Port=2
  1-D Lookup Table : Lookup_n-D  NumberOfTableDimensions=1  BreakpointsForDimension1=[1:7]  Table=[-0.05,0.01,.2,.2,.4,.8,1]  InternalRulePriority=Speed  RndMeth=Simplest
  Gain : Gain  Gain=.8
  Sin : Trigonometry
  Square : Math  Operator=square
  Switch : Switch  Criteria=u2 > Threshold  Threshold=7  SaturateOnIntegerOverflow=off  InputSameDT=off
  noseConeCd : Outport
  wires:
  noseConeType/1 -> 1-D Lookup Table/1, Switch/2
  joinAngle/1 -> Sin/1
  Sin/1 -> Square/1
  Square/1 -> Gain/1
  Gain/1 -> Switch/1
  1-D Lookup Table/1 -> Switch/3
  Switch/1 -> noseConeCd/1

== system FlightSim/Aerodyanmic Forces/Axial Drag/SkinFrictionCoefficeint(OLD)/If Action Subsystem ==
  Action Port : ActionPort  ActionPortLabel=if(u1 <= 10E4)
  Constant : Constant  Value=1.48E-2
  Cf : Outport
  wires:
  Constant/1 -> Cf/1

== system FlightSim/Aerodyanmic Forces/Axial Drag/SkinFrictionCoefficeint(OLD)/If Action Subsystem1 ==
  note: 10E4 < R < R_crit
  Re : Inport
  Action Port : ActionPort  ActionPortLabel=elseif(10E4 <= u1 <= u2)
  Constant2 : Constant  Value=5.6
  Constant3 : Constant
  Divide2 : Product  Inputs=*/
  Gain : Gain  Gain=1.5
  Log : Math  Operator=log
  Square : Math  Operator=square
  Subtract : Sum  Inputs=+-
  Cf : Outport
  wires:
  Subtract/1 -> Square/1
  Gain/1 -> Subtract/1
  Log/1 -> Gain/1
  Constant2/1 -> Subtract/2
  Divide2/1 -> Cf/1
  Square/1 -> Divide2/2
  Re/1 -> Log/1
  Constant3/1 -> Divide2/1

== system FlightSim/Aerodyanmic Forces/Axial Drag/SkinFrictionCoefficeint(OLD)/If Action Subsystem2 ==
  note: R> R_crit
  R_s : Inport
  rocketLength : Inport  Port=2
  Action Port : ActionPort  ActionPortLabel="elseif(  u1>u2)"
  Cf(R>R_Crit) : Product
  Constant4 : Constant  Value=.2
  Constant5 : Constant  Value=0.032
  Divide3 : Product  Inputs=*/
  Power1 : Math  Operator=pow
  Cf : Outport
  wires:
  Power1/1 -> Cf(R>R_Crit)/1
  Divide3/1 -> Power1/1
  Constant4/1 -> Power1/2
  Constant5/1 -> Cf(R>R_Crit)/2
  rocketLength/1 -> Divide3/2
  R_s/1 -> Divide3/1
  Cf(R>R_Crit)/1 -> Cf/1

== system FlightSim/Aerodyanmic Forces/Normal Force and Pitch Moment Calc/Cna of Fins/Case 1 ==
  rollAngle : Inport
  Action Port : ActionPort  ActionPortLabel=case [ 1 ]:
  Sin : Trigonometry
  Square5 : Math  Operator=square
  CNaTotal : Outport
  wires:
  Sin/1 -> Square5/1
  rollAngle/1 -> Sin/1
  Square5/1 -> CNaTotal/1

== system FlightSim/Aerodyanmic Forces/Normal Force and Pitch Moment Calc/Cna of Fins/Case 2 ==
  note: this is me guesing, but if 2 fins symmetric, one is 180 degrees from the other so you can find roll angle of the 2nd from the first easily
  rollAngle : Inport
  Action Port : ActionPort  ActionPortLabel=case [ 2 ]:
  Add : Sum
  Add1 : Sum
  Pi : Constant  Value=pi  OutDataTypeStr=Inherit: Inherit via back propagation
  Sin : Trigonometry
  Sin1 : Trigonometry
  Square1 : Math  Operator=square
  Square5 : Math  Operator=square
  CNaTotal : Outport
  wires:
  Add1/1 -> CNaTotal/1
  Sin/1 -> Square5/1
  rollAngle/1 -> Add/1, Sin/1
  Square5/1 -> Add1/1
  Pi/1 -> Add/2
  Sin1/1 -> Square1/1
  Add/1 -> Sin1/1
  Square1/1 -> Add1/2

== system FlightSim/Aerodyanmic Forces/Normal Force and Pitch Moment Calc/Cna of Fins/Case 3 ==
  note: OLD IMPLEMENTATION
  note: based on open rocket's implementation for Fin CNA, not rly sure why this is the case but wutever. I think theta is wind direction? it changes based on the change in wind direction in component analysis so im stickin with that
  rollAngle : Inport
  Action Port : ActionPort  ActionPortLabel=case [ 3 ]:
  Constant : Constant  Commented=on
  Constant1 : Constant  Commented=on  Value=-2/3
  Constant2 : Constant  Value=3/2
  Cos : Trigonometry  Commented=through  Operator=cos
  Gain : Gain  Commented=through  Gain=1.5
  Gain1 : Gain  Commented=through  Gain=.15
  Gain2 : Gain  Commented=through  Gain=1.5
  Pi : Constant  Commented=on  Value=pi  OutDataTypeStr=Inherit: Inherit via back propagation
  Product : Product  Commented=on
  Sin : Trigonometry  Commented=on
  Square : Math  Commented=through  Operator=square
  Square1 : Math  Commented=on  Operator=square
  Subtract : Sum  Commented=on  Inputs=+-
  Subtract1 : Sum  Commented=on  Inputs=+-
  CnaTotal : Outport
  wires:
  Constant/1 -> Subtract/1
  Gain/1 -> Cos/1
  Cos/1 -> Square/1
  Square/1 -> Gain1/1
  Gain1/1 -> Subtract/2
  Subtract/1 -> Gain2/1
  Pi/1 -> Product/2
  Product/1 -> Sin/1
  Sin/1 -> Square1/1
  rollAngle/1 -> Subtract1/1
  Subtract1/1 -> Product/1
  Constant1/1 -> Subtract1/2
  Constant2/1 -> CnaTotal/1

== system FlightSim/Aerodyanmic Forces/Normal Force and Pitch Moment Calc/Cna of Fins/Case 4 ==
  Action Port : ActionPort  ActionPortLabel=case [ 4 ]:
  Constant : Constant  Commented=on
  Constant1 : Constant  Commented=on  Value=-1/2
  Constant2 : Constant  Value=2
  Cos : Trigonometry  Commented=through
  Gain : Gain  Commented=through  Gain=2
  Gain1 : Gain  Commented=on  Gain=0.06
  Gain2 : Gain  Commented=through  Gain=2
  Pi : Constant  Commented=on  Value=pi  OutDataTypeStr=Inherit: Inherit via back propagation
  Product : Product  Commented=on
  Sin : Trigonometry  Commented=on
  Square : Math  Commented=on  Operator=square
  Square1 : Math  Commented=on  Operator=square
  Subtract : Sum  Commented=on  Inputs=+-
  Subtract1 : Sum  Commented=on  Inputs=+-
  CnaTotal : Outport
  wires:
  Constant/1 -> Subtract/1
  Gain/1 -> Cos/1
  Cos/1 -> Square/1
  Square/1 -> Gain1/1
  Gain1/1 -> Subtract/2
  Subtract/1 -> Gain2/1
  Pi/1 -> Product/2
  Constant1/1 -> Subtract1/1
  Sin/1 -> Square1/1
  Product/1 -> Sin/1
  Subtract1/1 -> Product/1
  Constant2/1 -> CnaTotal/1

== system FlightSim/Aerodyanmic Forces/Normal Force and Pitch Moment Calc/Cna of Fins/Case 5 ==
  Action Port : ActionPort  ActionPortLabel=case [ 5 ]:
  Constant : Constant  Value=2.37
  CnaTotal : Outport
  wires:
  Constant/1 -> CnaTotal/1

== system FlightSim/Aerodyanmic Forces/Normal Force and Pitch Moment Calc/Cna of Fins/Case 6 ==
  Action Port : ActionPort  ActionPortLabel=case [ 6 ]:
  Constant : Constant  Value=2.74
  CnaTotal : Outport
  wires:
  Constant/1 -> CnaTotal/1

== system FlightSim/Aerodyanmic Forces/Normal Force and Pitch Moment Calc/Cna of Fins/Case 7 ==
  Action Port : ActionPort  ActionPortLabel=case [ 7 ]:
  Constant : Constant  Value=2.99
  CnaTotal : Outport
  wires:
  Constant/1 -> CnaTotal/1

== system FlightSim/Aerodyanmic Forces/Normal Force and Pitch Moment Calc/Cna of Fins/Case 8 ==
  Action Port : ActionPort  ActionPortLabel=case [ 8 ]:
  Constant : Constant  Value=3.24
  CnaTotal : Outport
  wires:
  Constant/1 -> CnaTotal/1

== system FlightSim/Aerodyanmic Forces/Normal Force and Pitch Moment Calc/Cna of Fins/Case 9 ==
  Action Port : ActionPort  ActionPortLabel=default:
  Constant : Constant  Value=2
  CnaTotal : Outport
  wires:
  Constant/1 -> CnaTotal/1
